simulink model "two_adcs_rfsoc_if_calibrator"  (R2021a) (continued)
== system two_adcs_rfsoc_if_calibrator == (continued)
      mask DialogControl t224_DT_adc0_DataSettings Prompt: Data Settings
      mask DialogControl t224_DT_adc0_req_axis_clk_label Prompt: Required AXI4-Stream clock:
      mask DialogControl t224_DT_adc0_MixerSettings Prompt: MIxer Settings
      mask DialogControl t224_DT_adc0_AnalogSettings Prompt: Analog Settings
      mask DialogControl t224_DT_adc1 Prompt: ADC 1
      mask DialogControl t224_DT_adc1_DataSettings Prompt: Data Settings
      mask DialogControl t224_DT_adc1_req_axis_clk_label Prompt: Required AXI4-Stream clock:
      mask DialogControl t224_DT_adc1_MixerSettings Prompt: MIxer Settings
      mask DialogControl t224_DT_adc1_AnalogSettings Prompt: Analog Settings
      mask DialogControl Tile225_container Prompt: Tile 225
      mask DialogControl pll_clocking_config_225 Prompt: Clocking and PLL Configuration
      mask DialogControl t225_QuadTileConfig Prompt: Quad Tile Configuration
      mask DialogControl t225_ADCPair01 Prompt: ADC Pair 0,1
      mask DialogControl t225_QT_adc0 Prompt: ADC 0
      mask DialogControl t225_QT_adc0_DataSettings Prompt: Data Settings
      mask DialogControl t225_QT_adc0_req_axis_clk_label Prompt: Required AXI4-Stream clock:
      mask DialogControl t225_QT_adc0_MixerSettings Prompt: MIxer Settings
      mask DialogControl t225_QT_adc0_AnalogSettings Prompt: Analog Settings
      mask DialogControl t225_QT_adc1 Prompt: ADC 1
      mask DialogControl t225_QT_adc1_DataSettings Prompt: Data Settings
      mask DialogControl t225_QT_adc1_req_axis_clk_label Prompt: Required AXI4-Stream clock:
      mask DialogControl t225_QT_adc1_MixerSettings Prompt: MIxer Settings
      mask DialogControl t225_QT_adc1_AnalogSettings Prompt: Analog Settings
      mask DialogControl t225_ADCPair23 Prompt: ADC Pair 2,3
      mask DialogControl t225_QT_adc2 Prompt: ADC 2
      mask DialogControl t225_QT_adc2_DataSettings Prompt: Data Settings
      mask DialogControl t225_QT_adc2_req_axis_clk_label Prompt: Required AXI4-Stream clock:
      mask DialogControl t225_QT_adc2_MixerSettings Prompt: MIxer Settings
      mask DialogControl t225_QT_adc2_AnalogSettings Prompt: Analog Settings
      mask DialogControl t225_QT_adc3 Prompt: ADC 3
      mask DialogControl t225_QT_adc3_DataSettings Prompt: Data Settings
      mask DialogControl t225_QT_adc3_req_axis_clk_label Prompt: Required AXI4-Stream clock:
      mask DialogControl t225_QT_adc3_MixerSettings Prompt: MIxer Settings
      mask DialogControl t225_QT_adc3_AnalogSettings Prompt: Analog Settings
      mask DialogControl t225_DualTileConfig Prompt: Dual Tile Configuration
      mask DialogControl t225_DT_adc0 Prompt: ADC 0
      mask DialogControl t225_DT_adc0_DataSettings Prompt: Data Settings
      mask DialogControl t225_DT_adc0_req_axis_clk_label Prompt: Required AXI4-Stream clock:
      mask DialogControl t225_DT_adc0_MixerSettings Prompt: MIxer Settings
      mask DialogControl t225_DT_adc0_AnalogSettings Prompt: Analog Settings
      mask DialogControl t225_DT_adc1 Prompt: ADC 1
      mask DialogControl t225_DT_adc1_DataSettings Prompt: Data Settings
      mask DialogControl t225_DT_adc1_req_axis_clk_label Prompt: Required AXI4-Stream clock:
      mask DialogControl t225_DT_adc1_MixerSettings Prompt: MIxer Settings
      mask DialogControl t225_DT_adc1_AnalogSettings Prompt: Analog Settings
      mask DialogControl Tile226_container Prompt: Tile 226
      mask DialogControl pll_clocking_config_226 Prompt: Clocking and PLL Configuration
      mask DialogControl t226_QuadTileConfig Prompt: Quad Tile Configuration
      mask DialogControl t226_ADCPair01 Prompt: ADC Pair 0,1
      mask DialogControl t226_QT_adc0 Prompt: ADC 0
      mask DialogControl t226_QT_adc0_DataSettings Prompt: Data Settings
      mask DialogControl t226_QT_adc0_req_axis_clk_label Prompt: Required AXI4-Stream clock:
      mask DialogControl t226_QT_adc0_MixerSettings Prompt: MIxer Settings
      mask DialogControl t226_QT_adc0_AnalogSettings Prompt: Analog Settings
      mask DialogControl t226_QT_adc1 Prompt: ADC 1
      mask DialogControl t226_QT_adc1_DataSettings Prompt: Data Settings
      mask DialogControl t226_QT_adc1_req_axis_clk_label Prompt: Required AXI4-Stream clock:
      mask DialogControl t226_QT_adc1_MixerSettings Prompt: MIxer Settings
      mask DialogControl t226_QT_adc1_AnalogSettings Prompt: Analog Settings
      mask DialogControl t226_ADCPair23 Prompt: ADC Pair 2,3
      mask DialogControl t226_QT_adc2 Prompt: ADC 2
      mask DialogControl t226_QT_adc2_DataSettings Prompt: Data Settings
      mask DialogControl t226_QT_adc2_req_axis_clk_label Prompt: Required AXI4-Stream clock:
      mask DialogControl t226_QT_adc2_MixerSettings Prompt: MIxer Settings
      mask DialogControl t226_QT_adc2_AnalogSettings Prompt: Analog Settings
      mask DialogControl t226_QT_adc3 Prompt: ADC 3
      mask DialogControl t226_QT_adc3_DataSettings Prompt: Data Settings
      mask DialogControl t226_QT_adc3_req_axis_clk_label Prompt: Required AXI4-Stream clock:
      mask DialogControl t226_QT_adc3_MixerSettings Prompt: MIxer Settings
      mask DialogControl t226_QT_adc3_AnalogSettings Prompt: Analog Settings
      mask DialogControl t226_DualTileConfig Prompt: Dual Tile Configuration
      mask DialogControl t226_DT_adc0 Prompt: ADC 0
      mask DialogControl t226_DT_adc0_DataSettings Prompt: Data Settings
      mask DialogControl t226_DT_adc0_req_axis_clk_label Prompt: Required AXI4-Stream clock:
      mask DialogControl t226_DT_adc0_MixerSettings Prompt: MIxer Settings
      mask DialogControl t226_DT_adc0_AnalogSettings Prompt: Analog Settings
      mask DialogControl t226_DT_adc1 Prompt: ADC 1
      mask DialogControl t226_DT_adc1_DataSettings Prompt: Data Settings
      mask DialogControl t226_DT_adc1_req_axis_clk_label Prompt: Required AXI4-Stream clock:
      mask DialogControl t226_DT_adc1_MixerSettings Prompt: MIxer Settings
      mask DialogControl t226_DT_adc1_AnalogSettings Prompt: Analog Settings
      mask DialogControl Tile227_container Prompt: Tile 227
      mask DialogControl pll_clocking_config_227 Prompt: Clocking and PLL Configuration
      mask DialogControl t227_QuadTileConfig Prompt: Quad Tile Configuration
      mask DialogControl t227_ADCPair01 Prompt: ADC Pair 0,1
      mask DialogControl t227_QT_adc0 Prompt: ADC 0
      mask DialogControl t227_QT_adc0_DataSettings Prompt: Data Settings
      mask DialogControl t227_QT_adc0_req_axis_clk_label Prompt: Required AXI4-Stream clock:
      mask DialogControl t227_QT_adc0_MixerSettings Prompt: MIxer Settings
      mask DialogControl t227_QT_adc0_AnalogSettings Prompt: Analog Settings
      mask DialogControl t227_QT_adc1 Prompt: ADC 1
      mask DialogControl t227_QT_adc1_DataSettings Prompt: Data Settings
      mask DialogControl t227_QT_adc1_req_axis_clk_label Prompt: Required AXI4-Stream clock:
      mask DialogControl t227_QT_adc1_MixerSettings Prompt: MIxer Settings
      mask DialogControl t227_QT_adc1_AnalogSettings Prompt: Analog Settings
      mask DialogControl t227_ADCPair23 Prompt: ADC Pair 2,3
      mask DialogControl t227_QT_adc2 Prompt: ADC 2
      mask DialogControl t227_QT_adc2_DataSettings Prompt: Data Settings
      mask DialogControl t227_QT_adc2_req_axis_clk_label Prompt: Required AXI4-Stream clock:
      mask DialogControl t227_QT_adc2_MixerSettings Prompt: MIxer Settings
      mask DialogControl t227_QT_adc2_AnalogSettings Prompt: Analog Settings
      mask DialogControl t227_QT_adc3 Prompt: ADC 3
      mask DialogControl t227_QT_adc3_DataSettings Prompt: Data Settings
      mask DialogControl t227_QT_adc3_req_axis_clk_label Prompt: Required AXI4-Stream clock:
      mask DialogControl t227_QT_adc3_MixerSettings Prompt: MIxer Settings
      mask DialogControl t227_QT_adc3_AnalogSettings Prompt: Analog Settings
      mask DialogControl t227_DualTileConfig Prompt: Dual Tile Configuration
      mask DialogControl t227_DT_adc0 Prompt: ADC 0
      mask DialogControl t227_DT_adc0_DataSettings Prompt: Data Settings
      mask DialogControl t227_DT_adc0_req_axis_clk_label Prompt: Required AXI4-Stream clock:
      mask DialogControl t227_DT_adc0_MixerSettings Prompt: MIxer Settings
      mask DialogControl t227_DT_adc0_AnalogSettings Prompt: Analog Settings
      mask DialogControl t227_DT_adc1 Prompt: ADC 1
      mask DialogControl t227_DT_adc1_DataSettings Prompt: Data Settings
      mask DialogControl t227_DT_adc1_req_axis_clk_label Prompt: Required AXI4-Stream clock:
      mask DialogControl t227_DT_adc1_MixerSettings Prompt: MIxer Settings
      mask DialogControl t227_DT_adc1_AnalogSettings Prompt: Analog Settings
      mask DialogControl DACTileSelect Prompt: DAC Tiles
      mask DialogControl Tile228_container Prompt: Tile 228
      mask DialogControl pll_clocking_config_228 Prompt: Clocking and PLL Configuration
      mask DialogControl t228_QuadTileConfig Prompt: Quad Tile Configuration
      mask DialogControl t228_DACPair01 Prompt: DAC Pair 0,1
      mask DialogControl t228_QT_dac0 Prompt: DAC 0
      mask DialogControl t228_QT_dac0_DataSettings Prompt: Data Settings
      mask DialogControl t228_QT_dac0_req_axis_clk_label Prompt: Required AXI4-Stream clock:
      mask DialogControl t228_QT_dac0_MixerSettings Prompt: MIxer Settings
      mask DialogControl t228_QT_dac0_AnalogSettings Prompt: Analog Settings
      mask DialogControl t228_QT_dac1 Prompt: DAC 1
      mask DialogControl t228_QT_dac1_DataSettings Prompt: Data Settings
      mask DialogControl t228_QT_dac1_req_axis_clk_label Prompt: Required AXI4-Stream clock:
      mask DialogControl t228_QT_dac1_MixerSettings Prompt: MIxer Settings
      mask DialogControl t228_QT_dac1_AnalogSettings Prompt: Analog Settings
      mask DialogControl t228_DACPair23 Prompt: DAC Pair 2,3
      mask DialogControl t228_QT_dac2 Prompt: DAC 2
      mask DialogControl t228_QT_dac2_DataSettings Prompt: Data Settings
      mask DialogControl t228_QT_dac2_req_axis_clk_label Prompt: Required AXI4-Stream clock:
      mask DialogControl t228_QT_dac2_MixerSettings Prompt: MIxer Settings
      mask DialogControl t228_QT_dac2_AnalogSettings Prompt: Analog Settings
      mask DialogControl t228_QT_dac3 Prompt: DAC 3
      mask DialogControl t228_QT_dac3_DataSettings Prompt: Data Settings
      mask DialogControl t228_QT_dac3_req_axis_clk_label Prompt: Required AXI4-Stream clock:
      mask DialogControl t228_QT_dac3_MixerSettings Prompt: MIxer Settings
      mask DialogControl t228_QT_dac3_AnalogSettings Prompt: Analog Settings
      mask DialogControl t228_DualTileConfig Prompt: Dual Tile Configuration
      mask DialogControl t228_DACPair01_DT Prompt: DAC Pair 0,1
      mask DialogControl t228_DT_dac0 Prompt: DAC 0
      mask DialogControl t228_DT_dac0_DataSettings Prompt: Data Settings
      mask DialogControl t228_DT_dac0_req_axis_clk_label Prompt: Required AXI4-Stream clock:
      mask DialogControl t228_DT_dac0_MixerSettings Prompt: MIxer Settings
      mask DialogControl t228_DT_dac0_AnalogSettings Prompt: Analog Settings
      mask DialogControl t228_DT_dac1 Prompt: DAC 1
      mask DialogControl t228_DT_dac1_DataSettings Prompt: Data Settings
      mask DialogControl t228_DT_dac1_req_axis_clk_label Prompt: Required AXI4-Stream clock:
      mask DialogControl t228_DT_dac1_MixerSettings Prompt: MIxer Settings
      mask DialogControl t228_DT_dac1_AnalogSettings Prompt: Analog Settings
      mask DialogControl t228_DACPair23_DT Prompt: DAC Pair 2,3
      mask DialogControl t228_DT_dac2 Prompt: DAC 2
      mask DialogControl t228_DT_dac2_DataSettings Prompt: Data Settings
      mask DialogControl t228_DT_dac2_req_axis_clk_label Prompt: Required AXI4-Stream clock:
      mask DialogControl t228_DT_dac2_MixerSettings Prompt: MIxer Settings
      mask DialogControl t228_DT_dac2_AnalogSettings Prompt: Analog Settings
      mask DialogControl t228_DT_dac3 Prompt: DAC 3
      mask DialogControl t228_DT_dac3_DataSettings Prompt: Data Settings
      mask DialogControl t228_DT_dac3_req_axis_clk_label Prompt: Required AXI4-Stream clock:
      mask DialogControl t228_DT_dac3_MixerSettings Prompt: MIxer Settings
      mask DialogControl t228_DT_dac3_AnalogSettings Prompt: Analog Settings
      mask DialogControl Tile229_container Prompt: Tile 229
      mask DialogControl pll_clocking_config_229 Prompt: Clocking and PLL Configuration
      mask DialogControl t229_QuadTileConfig Prompt: Quad Tile Configuration
      mask DialogControl t229_DACPair01 Prompt: DAC Pair 0,1
      mask DialogControl t229_QT_dac0 Prompt: DAC 0
      mask DialogControl t229_QT_dac0_DataSettings Prompt: Data Settings
      mask DialogControl t229_QT_dac0_req_axis_clk_label Prompt: Required AXI4-Stream clock:
      mask DialogControl t229_QT_dac0_MixerSettings Prompt: MIxer Settings
      mask DialogControl t229_QT_dac0_AnalogSettings Prompt: Analog Settings
      mask DialogControl t229_QT_dac1 Prompt: DAC 1
      mask DialogControl t229_QT_dac1_DataSettings Prompt: Data Settings
      mask DialogControl t229_QT_dac1_req_axis_clk_label Prompt: Required AXI4-Stream clock:
      mask DialogControl t229_QT_dac1_MixerSettings Prompt: MIxer Settings
      mask DialogControl t229_QT_dac1_AnalogSettings Prompt: Analog Settings
      mask DialogControl t229_DACPair23 Prompt: DAC Pair 2,3
      mask DialogControl t229_QT_dac2 Prompt: DAC 2
      mask DialogControl t229_QT_dac2_DataSettings Prompt: Data Settings
      mask DialogControl t229_QT_dac2_req_axis_clk_label Prompt: Required AXI4-Stream clock:
      mask DialogControl t229_QT_dac2_MixerSettings Prompt: MIxer Settings
      mask DialogControl t229_QT_dac2_AnalogSettings Prompt: Analog Settings
      mask DialogControl t229_QT_dac3 Prompt: DAC 3
      mask DialogControl t229_QT_dac3_DataSettings Prompt: Data Settings
      mask DialogControl t229_QT_dac3_req_axis_clk_label Prompt: Required AXI4-Stream clock:
      mask DialogControl t229_QT_dac3_MixerSettings Prompt: MIxer Settings
      mask DialogControl t229_QT_dac3_AnalogSettings Prompt: Analog Settings
      mask DialogControl t229_DualTileConfig Prompt: Dual Tile Configuration
      mask DialogControl t229_DACPair01_DT Prompt: DAC Pair 0,1
      mask DialogControl t229_DT_dac0 Prompt: DAC 0
      mask DialogControl t229_DT_dac0_DataSettings Prompt: Data Settings
      mask DialogControl t229_DT_dac0_req_axis_clk_label Prompt: Required AXI4-Stream clock:
      mask DialogControl t229_DT_dac0_MixerSettings Prompt: MIxer Settings
      mask DialogControl t229_DT_dac0_AnalogSettings Prompt: Analog Settings
      mask DialogControl t229_DT_dac1 Prompt: DAC 1
      mask DialogControl t229_DT_dac1_DataSettings Prompt: Data Settings
      mask DialogControl t229_DT_dac1_req_axis_clk_label Prompt: Required AXI4-Stream clock:
      mask DialogControl t229_DT_dac1_MixerSettings Prompt: MIxer Settings
      mask DialogControl t229_DT_dac1_AnalogSettings Prompt: Analog Settings
      mask DialogControl t229_DACPair23_DT Prompt: DAC Pair 2,3
      mask DialogControl t229_DT_dac2 Prompt: DAC 2
      mask DialogControl t229_DT_dac2_DataSettings Prompt: Data Settings
      mask DialogControl t229_DT_dac2_req_axis_clk_label Prompt: Required AXI4-Stream clock:
      mask DialogControl t229_DT_dac2_MixerSettings Prompt: MIxer Settings
      mask DialogControl t229_DT_dac2_AnalogSettings Prompt: Analog Settings
      mask DialogControl t229_DT_dac3 Prompt: DAC 3
      mask DialogControl t229_DT_dac3_DataSettings Prompt: Data Settings
      mask DialogControl t229_DT_dac3_req_axis_clk_label Prompt: Required AXI4-Stream clock:
      mask DialogControl t229_DT_dac3_MixerSettings Prompt: MIxer Settings
      mask DialogControl t229_DT_dac3_AnalogSettings Prompt: Analog Settings
      mask DialogControl Tile230_container Prompt: Tile 230
      mask DialogControl pll_clocking_config_230 Prompt: Clocking and PLL Configuration
      mask DialogControl t230_QuadTileConfig Prompt: Quad Tile Configuration
      mask DialogControl t230_DACPair01 Prompt: DAC Pair 0,1
      mask DialogControl t230_QT_dac0 Prompt: DAC 0
      mask DialogControl t230_QT_dac0_DataSettings Prompt: Data Settings
      mask DialogControl t230_QT_dac0_req_axis_clk_label Prompt: Required AXI4-Stream clock:
      mask DialogControl t230_QT_dac0_MixerSettings Prompt: MIxer Settings
      mask DialogControl t230_QT_dac0_AnalogSettings Prompt: Analog Settings
      mask DialogControl t230_QT_dac1 Prompt: DAC 1
      mask DialogControl t230_QT_dac1_DataSettings Prompt: Data Settings
      mask DialogControl t230_QT_dac1_req_axis_clk_label Prompt: Required AXI4-Stream clock:
      mask DialogControl t230_QT_dac1_MixerSettings Prompt: MIxer Settings
      mask DialogControl t230_QT_dac1_AnalogSettings Prompt: Analog Settings
      mask DialogControl t230_DACPair23 Prompt: DAC Pair 2,3
      mask DialogControl t230_QT_dac2 Prompt: DAC 2
      mask DialogControl t230_QT_dac2_DataSettings Prompt: Data Settings
      mask DialogControl t230_QT_dac2_req_axis_clk_label Prompt: Required AXI4-Stream clock:
      mask DialogControl t230_QT_dac2_MixerSettings Prompt: MIxer Settings
      mask DialogControl t230_QT_dac2_AnalogSettings Prompt: Analog Settings
      mask DialogControl t230_QT_dac3 Prompt: DAC 3
      mask DialogControl t230_QT_dac3_DataSettings Prompt: Data Settings
      mask DialogControl t230_QT_dac3_req_axis_clk_label Prompt: Required AXI4-Stream clock:
      mask DialogControl t230_QT_dac3_MixerSettings Prompt: MIxer Settings
      mask DialogControl t230_QT_dac3_AnalogSettings Prompt: Analog Settings
      mask DialogControl t230_DualTileConfig Prompt: Dual Tile Configuration
      mask DialogControl Control58 Prompt: Tile 230 does not exist for DT configs
      mask DialogControl Tile231_container Prompt: Tile 231
      mask DialogControl pll_clocking_config_231 Prompt: Clocking and PLL Configuration
      mask DialogControl t231_QuadTileConfig Prompt: Quad Tile Configuration
      mask DialogControl t231_DACPair01 Prompt: DAC Pair 0,1
      mask DialogControl t231_QT_dac0 Prompt: DAC 0
      mask DialogControl t231_QT_dac0_DataSettings Prompt: Data Settings
      mask DialogControl t231_QT_dac0_req_axis_clk_label Prompt: Required AXI4-Stream clock:
      mask DialogControl t231_QT_dac0_MixerSettings Prompt: MIxer Settings
      mask DialogControl t231_QT_dac0_AnalogSettings Prompt: Analog Settings
      mask DialogControl t231_QT_dac1 Prompt: DAC 1
      mask DialogControl t231_QT_dac1_DataSettings Prompt: Data Settings
      mask DialogControl t231_QT_dac1_req_axis_clk_label Prompt: Required AXI4-Stream clock:
      mask DialogControl t231_QT_dac1_MixerSettings Prompt: MIxer Settings
      mask DialogControl t231_QT_dac1_AnalogSettings Prompt: Analog Settings
      mask DialogControl t231_DACPair23 Prompt: DAC Pair 2,3
      mask DialogControl t231_QT_dac2 Prompt: DAC 2
      mask DialogControl t231_QT_dac2_DataSettings Prompt: Data Settings
      mask DialogControl t231_QT_dac2_req_axis_clk_label Prompt: Required AXI4-Stream clock:
      mask DialogControl t231_QT_dac2_MixerSettings Prompt: MIxer Settings
      mask DialogControl t231_QT_dac2_AnalogSettings Prompt: Analog Settings
      mask DialogControl t231_QT_dac3 Prompt: DAC 3
      mask DialogControl t231_QT_dac3_DataSettings Prompt: Data Settings
      mask DialogControl t231_QT_dac3_req_axis_clk_label Prompt: Required AXI4-Stream clock:
      mask DialogControl t231_QT_dac3_MixerSettings Prompt: MIxer Settings
      mask DialogControl t231_QT_dac3_AnalogSettings Prompt: Analog Settings
      mask DialogControl t231_DualTileConfig Prompt: Dual Tile Configuration
      mask DialogControl Control59 Prompt: Tile 231 does not exist for DT configs
      mask DialogControl SystemClocking Prompt: System Clocking
      mask DialogControl ADCClocking Prompt: ADC Tile Clocking
      mask DialogControl t224_clock_config Prompt: ADC Tile 0
      mask DialogControl t225_clock_config Prompt: ADC Tile 1
      mask DialogControl t226_clock_config Prompt: ADC Tile 2
      mask DialogControl t227_clock_config Prompt: ADC Tile 3
      mask DialogControl DACClocking Prompt: DAC Tile Clocking
      mask DialogControl t228_clock_config Prompt: DAC Tile 0
      mask DialogControl t229_clock_config Prompt: DAC Tile 1
      mask DialogControl t230_clock_config Prompt: DAC Tile 2
      mask DialogControl t231_clock_config Prompt: DAC Tile 3
  snap_trig : Subsystem  Tag=xps:sw_reg  AncestorBlock=xps_library/Memory/software_register  mask(swreg){io_dir=From Processor, io_delay=0, init_val=dec2hex(0), sample_period=1, names=reg, bitwidths=32, bin_pts=0, arith_types=2, sim_port=off, show_format=on}
      mask prompts: io_dir "I/O direction"; io_delay "I/O delay"; init_val "Initial Value"; sample_period "Sample period"; names "Bitfield names [msb...lsb]"; bitwidths "Bitfield widths"; bin_pts "Bitfield binary pts"; arith_types "Bitfield types, ufix=0, fix=1, bool=2"; sim_port "Provide sim input/output?"; show_format "Print format string?"
      mask help: A 32-bit software-accessible register. Can be divided into bitfields of varying widths and types using the fields in the block mask.
      mask init:
      | swreg_init(gcb)
      settings: AttributesFormatString=1input:b = 32 bits
      mask TypeOptions io_dir: To Processor | From Processor
      mask Callback io_dir:
      | mask_enables = get_param(gcb, 'MaskEnables');
      | mask_names = get_param(gcb, 'MaskNames');
      | if strcmp(get_param(gcb, 'io_dir'), 'To Processor'),
      |     mask_enables{ismember(mask_names, 'sample_period')} = 'off';
      | else
      |     mask_enables{ismember(mask_names, 'sample_period')} = 'on';
      | end
      | set_param(gcb, 'MaskEnables', mask_enables);
      mask DialogControl ParameterTabVar0 Prompt: Setup
  sync_gen : Subsystem  AncestorBlock=casper_library_misc/sync_gen  mask(sync_gen){sim_acc_len=1, fft_size=2048, fft_simult_inputs=8, pfb_fir_taps=3, reorder_vec=[2,2,8], scale=4096}
      mask prompts: sim_acc_len "Simulation Accumulation Length"; fft_size "FFT Size"; fft_simult_inputs "Simultaneous Inputs (FFT)"; pfb_fir_taps "Taps in pfb_fir (use 1 if no pfb_fir)"; reorder_vec "Reorder Orders (e.g. [2 2 2])"
      mask help:
      | This block computes the sync period based on system parameters as
      | described in Casper memo.  Detailed descritions of the block's
      | variables can be found in the 'Block Documentation' section of
      | the CASPER wiki.
      mask init:
      | sync_gen_init(gcb, ...
      |     'sim_acc_len', sim_acc_len, ...
      |     'fft_size', fft_size, ...
      |     'fft_simult_inputs', fft_simult_inputs,...
      |     'pfb_fir_taps', pfb_fir_taps, ...
      |     'reorder_vec', reorder_vec, ...
      |     'scale', scale);
      settings: AttributesFormatString=sim_sync_period=25165824
      mask Help:
      | SyncPeriod=LCM(reorder_vec)*(acc_num)*(pfb_fir_len)*(fft_size/fft_simult_inputs)
      | 
      | See CASPER sync pulse memo for more details.
  wires:
  Constant2/1 -> rfdc/1(m00_axis_tdata_sim), rfdc/2(m01_axis_tdata_sim), rfdc/3(m02_axis_tdata_sim), rfdc/4(m03_axis_tdata_sim), rfdc/5(m10_axis_tdata_sim), rfdc/6(m12_axis_tdata_sim), rfdc/8(m21_axis_tdata_sim), rfdc/12(m32_axis_tdata_sim), rfdc/11(m30_axis_tdata_sim), rfdc/10(m23_axis_tdata_sim), rfdc/9(m22_axis_tdata_sim), rfdc/7(m20_axis_tdata_sim)
  snap_trig/1(in_reg) -> [snap_trig]
  pfb_fir0/1(sync_out) -> fft0/1(sync)
  pfb_fir0/2(pol1_out1) -> fft0/3(in00)
  pfb_fir0/3(pol1_out2) -> fft0/4(in01)
  pfb_fir0/4(pol1_out3) -> fft0/5(in02)
  pfb_fir0/5(pol1_out4) -> fft0/6(in03)
  pfb_fir0/6(pol1_out5) -> fft0/7(in04)
  pfb_fir0/7(pol1_out6) -> fft0/8(in05)
  pfb_fir0/8(pol1_out7) -> fft0/9(in06)
  pfb_fir0/9(pol1_out8) -> fft0/10(in07)
  pre_power_delay0/2(dout0) -> pre_correlation_delay0/2(din0), power0/2(din0), debug_sys/3(fft0_0), calibrator_1/2(fft0_0), calibrator_0/2(fft0_0)
  pre_power_delay0/3(dout1) -> pre_correlation_delay0/3(din1), power0/3(din1), calibrator_1/3(fft0_1), calibrator_0/3(fft0_1)
  pre_power_delay0/4(dout2) -> calibrator_1/4(fft0_2), calibrator_0/4(fft0_2), pre_correlation_delay0/4(din2), power0/4(din2)
  pre_power_delay0/5(dout3) -> calibrator_1/5(fft0_3), calibrator_0/5(fft0_3), pre_correlation_delay0/5(din3), power0/5(din3)
  [cnt_rst] -> acc_cntrl0/3(rst)
  [acc_len_cal] -> acc_cntrl0/1(acc_len)
  post_power_delay0/1(sync_out) -> acc_cntrl0/2(sync)
  pipeline8/1(q) -> [acc_len_cal]
  acc_len_cal/1(in_reg) -> pipeline8/1(d)
  pipeline1/1(q) -> [cnt_rst]
  cnt_rst/1(in_reg) -> pipeline1/1(d)
  acc_cntrl0/1(new_acc) -> counter0/1, accs0/1(new_acc)
  post_power_delay0/2(dout0) -> Delay/1
  post_power_delay0/3(dout1) -> Delay1/1
  post_power_delay0/5(dout3) -> Delay3/1
  post_power_delay0/4(dout2) -> Delay2/1
  Delay/1 -> accs0/2(din)
  Delay1/1 -> accs0/3(din1)
  Delay2/1 -> accs0/4(din2)
  Delay3/1 -> accs0/5(din3)
  accs0/1(valid) -> counter0/2, pre_bram_delay0/2(valid_in)
  accs0/2(dout0) -> pre_bram_delay0/3(din0)
  accs0/3(dout1) -> pre_bram_delay0/4(din1)
  accs0/4(dout2) -> pre_bram_delay0/5(din2)
  accs0/5(dout3) -> pre_bram_delay0/6(din3)
  counter0/1 -> pre_bram_delay0/1(addr_in)
  pre_bram_delay0/1(addr_out) -> adc0_0/1(addr), adc0_1/1(addr), adc0_2/1(addr), adc0_7/1(addr), adc0_6/1(addr), adc0_5/1(addr), adc0_4/1(addr), adc0_3/1(addr)
  pre_bram_delay0/3(dout0) -> adc0_0/2(data_in)
  pre_bram_delay0/2(valid_out) -> adc0_7/3(we), adc0_6/3(we), adc0_5/3(we), adc0_4/3(we), adc0_3/3(we), adc0_2/3(we), adc0_1/3(we), adc0_0/3(we)
  pre_bram_delay0/4(dout1) -> adc0_1/2(data_in)
  pre_bram_delay0/5(dout2) -> adc0_2/2(data_in)
  pre_bram_delay0/6(dout3) -> adc0_3/2(data_in)
  adc0_0/1(data_out) -> Terminator3/1
  adc0_1/1(data_out) -> Terminator4/1
  adc0_2/1(data_out) -> Terminator5/1
  adc0_3/1(data_out) -> Terminator6/1
  power0/1(sync_out) -> power_convert0/1(sync_in)
  power0/2(dout0) -> power_convert0/2(din0)
  power0/3(dout1) -> power_convert0/3(din1)
  power0/4(dout2) -> power_convert0/4(din2)
  power0/5(dout3) -> power_convert0/5(din3)
  power_convert0/1(sync_out) -> post_power_delay0/1(sync_in)
  power_convert0/2(dout0) -> post_power_delay0/2(din0)
  power_convert0/3(dout1) -> post_power_delay0/3(din1)
  power_convert0/4(dout2) -> post_power_delay0/4(din2)
  power_convert0/5(dout3) -> post_power_delay0/5(din3)
  Constant6/1 -> [fft_shift]
  [fft_shift] -> fft0/2(shift)
  rfdc/1(m00_axis_tdata) -> adcsnap0/1(din_real), bus_create/1(in1)
  rfdc/2(m01_axis_tdata) -> adcsnap0/2(din_imag), bus_create/2(in2)
  bus_create/1(bus_out) -> munge1/1(din)
  munge1/1(dout) -> [m00]
  munge2/1(dout) -> [m02]
  bus_create1/1(bus_out) -> munge2/1(din)
  rfdc/3(m02_axis_tdata) -> bus_create1/1(in1), adcsnap1/1(din_real)
  rfdc/4(m03_axis_tdata) -> adcsnap1/2(din_imag), bus_create1/2(in2)
  bus_create3/1(bus_out) -> munge4/1(din)
  bus_create2/1(bus_out) -> munge3/1(din)
  rfdc/7(m20_axis_tdata) -> bus_create2/1(in1)
  rfdc/9(m22_axis_tdata) -> bus_create3/1(in1)
  rfdc/10(m23_axis_tdata) -> bus_create3/2(in2)
  rfdc/8(m21_axis_tdata) -> bus_create2/2(in2)
  munge3/1(dout) -> [m20]
  fft0/1(sync_out) -> pre_power_delay0/1(sync_in)
  fft0/2(out00) -> pre_power_delay0/2(din0)
  fft0/3(out01) -> pre_power_delay0/3(din1)
  fft0/4(out02) -> pre_power_delay0/4(din2)
  fft0/5(out03) -> pre_power_delay0/5(din3)
  fft0/6(out04) -> pre_power_delay0/6(din4)
  fft0/7(out05) -> pre_power_delay0/7(din5)
  fft0/8(out06) -> pre_power_delay0/8(din6)
  fft0/9(out07) -> pre_power_delay0/9(din7)
  pre_power_delay0/6(dout4) -> calibrator_1/6(fft0_4), calibrator_0/6(fft0_4), pre_correlation_delay0/6(din4), power0/6(din4)
  pre_power_delay0/7(dout5) -> calibrator_1/7(fft0_5), calibrator_0/7(fft0_5), pre_correlation_delay0/7(din5), power0/7(din5)
  pre_power_delay0/8(dout6) -> calibrator_1/8(fft0_6), calibrator_0/8(fft0_6), pre_correlation_delay0/8(din6), power0/8(din6)
  pre_power_delay0/9(dout7) -> calibrator_1/9(fft0_7), calibrator_0/9(fft0_7), pre_correlation_delay0/9(din7), power0/9(din7)
  power0/6(dout4) -> power_convert0/6(din4)
  power0/7(dout5) -> power_convert0/7(din5)
  power0/8(dout6) -> power_convert0/8(din6)
  power0/9(dout7) -> power_convert0/9(din7)
  power_convert0/6(dout4) -> post_power_delay0/6(din4)
  power_convert0/7(dout5) -> post_power_delay0/7(din5)
  power_convert0/8(dout6) -> post_power_delay0/8(din6)
  power_convert0/9(dout7) -> post_power_delay0/9(din7)
  Delay16/1 -> accs0/6(din4)
  post_power_delay0/6(dout4) -> Delay16/1
  post_power_delay0/7(dout5) -> Delay17/1
  post_power_delay0/8(dout6) -> Delay18/1
  post_power_delay0/9(dout7) -> Delay19/1
  Delay17/1 -> accs0/7(din5)
  Delay18/1 -> accs0/8(din6)
  Delay19/1 -> accs0/9(din7)
  accs0/6(dout4) -> pre_bram_delay0/7(din4)
  accs0/7(dout5) -> pre_bram_delay0/8(din5)
  accs0/8(dout6) -> pre_bram_delay0/9(din6)
  accs0/9(dout7) -> pre_bram_delay0/10(din7)
  adc0_7/1(data_out) -> Terminator23/1
  adc0_5/1(data_out) -> Terminator21/1
  adc0_6/1(data_out) -> Terminator22/1
  adc0_4/1(data_out) -> Terminator20/1
  pre_bram_delay0/7(dout4) -> adc0_4/2(data_in)
  pre_bram_delay0/8(dout5) -> adc0_5/2(data_in)
  pre_bram_delay0/9(dout6) -> adc0_6/2(data_in)
  pre_bram_delay0/10(dout7) -> adc0_7/2(data_in)
  pfb_fir1/4(pol1_out3) -> fft1/5(in02)
  fft1/7(out05) -> pre_power_delay1/7(din5)
  fft1/4(out02) -> pre_power_delay1/4(din2)
  pfb_fir1/5(pol1_out4) -> fft1/6(in03)
  pre_power_delay1/4(dout2) -> calibrator_1/12(fft1_2), calibrator_0/12(fft1_2), power1/4(din2), conjugation0/3(z2)
  pfb_fir1/1(sync_out) -> fft1/1(sync)
  pre_power_delay1/9(dout7) -> calibrator_1/17(fft1_7), calibrator_0/17(fft1_7), power1/9(din7), conjugation0/8(z7)
  pre_power_delay1/6(dout4) -> calibrator_1/14(fft1_4), calibrator_0/14(fft1_4), power1/6(din4), conjugation0/5(z4)
  pre_power_delay1/3(dout1) -> calibrator_0/11(fft1_1), calibrator_1/11(fft1_1), power1/3(din1), conjugation0/2(z1)
  fft1/5(out03) -> pre_power_delay1/5(din3)
  fft1/1(sync_out) -> pre_power_delay1/1(sync_in)
  pfb_fir1/9(pol1_out8) -> fft1/10(in07)
  pfb_fir1/6(pol1_out5) -> fft1/7(in04)
  fft1/2(out00) -> pre_power_delay1/2(din0)
  pfb_fir1/8(pol1_out7) -> fft1/9(in06)
  fft1/8(out06) -> pre_power_delay1/8(din6)
  pre_power_delay1/5(dout3) -> calibrator_1/13(fft1_3), calibrator_0/13(fft1_3), power1/5(din3), conjugation0/4(z3)
  pre_power_delay1/8(dout6) -> calibrator_1/16(fft1_6), calibrator_0/16(fft1_6), power1/8(din6), conjugation0/7(z6)
  pre_power_delay1/2(dout0) -> power1/2(din0), conjugation0/1(z), debug_sys/4(fft1_0), calibrator_1/10(fft1_0), calibrator_0/10(fft1_0)
  pfb_fir1/3(pol1_out2) -> fft1/4(in01)
  fft1/9(out07) -> pre_power_delay1/9(din7)
  pfb_fir1/2(pol1_out1) -> fft1/3(in00)
  pre_power_delay1/7(dout5) -> calibrator_1/15(fft1_5), calibrator_0/15(fft1_5), power1/7(din5), conjugation0/6(z5)
  fft1/6(out04) -> pre_power_delay1/6(din4)
  fft1/3(out01) -> pre_power_delay1/3(din1)
  pre_power_delay1/1(sync_out) -> power1/1(sync_in)
  pfb_fir1/7(pol1_out6) -> fft1/8(in05)
  [fft_shift] -> fft1/2(shift)
  munge4/1(dout) -> [m22]
  pre_correlation_delay0/1(sync_out) -> correlation_mults0/1(sync_in)
  pre_correlation_delay0/2(dout0) -> correlation_mults0/2(adc0_0)
  pre_correlation_delay0/3(dout1) -> correlation_mults0/3(adc0_1)
  pre_correlation_delay0/4(dout2) -> correlation_mults0/4(adc0_2)
  pre_correlation_delay0/5(dout3) -> correlation_mults0/5(adc0_3)
  pre_correlation_delay0/6(dout4) -> correlation_mults0/6(adc0_4)
  pre_correlation_delay0/7(dout5) -> correlation_mults0/7(adc0_5)
  pre_correlation_delay0/8(dout6) -> correlation_mults0/8(adc0_6)
  pre_correlation_delay0/9(dout7) -> correlation_mults0/9(adc0_7)
  conjugation0/1(z*) -> correlation_mults0/10(adc1_0)
  conjugation0/2(z*1) -> correlation_mults0/11(adc1_1)
  conjugation0/3(z*2) -> correlation_mults0/12(adc1_2)
  conjugation0/4(z*3) -> correlation_mults0/13(adc1_3)
  conjugation0/5(z*4) -> correlation_mults0/14(adc1_4)
  conjugation0/6(z*5) -> correlation_mults0/15(adc1_5)
  conjugation0/7(z*6) -> correlation_mults0/16(adc1_6)
  conjugation0/8(z*7) -> correlation_mults0/17(adc1_7)
  correlation_mults0/4(corr2) -> correlation_conv0/4(din2)
  correlation_mults0/3(corr1) -> correlation_conv0/3(din1)
  correlation_mults0/5(corr3) -> correlation_conv0/5(din3)
  correlation_mults0/2(corr0) -> correlation_conv0/2(din0)
  correlation_mults0/6(corr4) -> correlation_conv0/6(din4)
  correlation_mults0/1(sync_out) -> correlation_conv0/1(sync_in)
  correlation_mults0/7(corr5) -> correlation_conv0/7(din5)
  correlation_mults0/8(corr6) -> correlation_conv0/8(din6)
  correlation_mults0/9(corr7) -> correlation_conv0/9(din7)
  correlation_conv0/1(sync_out) -> post_correlation_delay0/1(sync_in)
  correlation_conv0/2(dout0) -> post_correlation_delay0/2(din0)
  correlation_conv0/3(dout1) -> post_correlation_delay0/3(din1)
  correlation_conv0/4(dout2) -> post_correlation_delay0/4(din2)
  correlation_conv0/5(dout3) -> post_correlation_delay0/5(din3)
  correlation_conv0/6(dout4) -> post_correlation_delay0/6(din4)
  correlation_conv0/7(dout5) -> post_correlation_delay0/7(din5)
  correlation_conv0/8(dout6) -> post_correlation_delay0/8(din6)
  correlation_conv0/9(dout7) -> post_correlation_delay0/9(din7)
  correlation_accs0/8(dout6) -> pre_bram_delay4/9(din6)
  Delay36/1 -> correlation_accs0/8(din6)
  post_correlation_delay0/3(dout1) -> Delay33/1
  counter4/1 -> pre_bram_delay4/1(addr_in)
  Delay33/1 -> correlation_accs0/3(din1)
  post_correlation_delay0/2(dout0) -> Delay32/1
  correlation_accs0/1(valid) -> counter4/2, pre_bram_delay4/2(valid_in)
  Delay34/1 -> correlation_accs0/6(din4)
  correlation_accs0/9(dout7) -> pre_bram_delay4/10(din7)
  post_correlation_delay0/5(dout3) -> Delay39/1
  correlation_accs0/2(dout0) -> pre_bram_delay4/3(din0)
  correlation_accs0/5(dout3) -> pre_bram_delay4/6(din3)
  post_correlation_delay0/8(dout6) -> Delay36/1
  post_correlation_delay0/9(dout7) -> Delay37/1
  post_correlation_delay0/1(sync_out) -> acc_cntrl4/2(sync)
  [cnt_rst] -> acc_cntrl4/3(rst)
  correlation_accs0/6(dout4) -> pre_bram_delay4/7(din4)
  Delay37/1 -> correlation_accs0/9(din7)
  correlation_accs0/4(dout2) -> pre_bram_delay4/5(din2)
  correlation_accs0/3(dout1) -> pre_bram_delay4/4(din1)
  post_correlation_delay0/7(dout5) -> Delay35/1
  correlation_accs0/7(dout5) -> pre_bram_delay4/8(din5)
  [acc_len_cal] -> acc_cntrl4/1(acc_len)
  post_correlation_delay0/6(dout4) -> Delay34/1
  post_correlation_delay0/4(dout2) -> Delay38/1
  Delay32/1 -> correlation_accs0/2(din)
  Delay38/1 -> correlation_accs0/4(din2)
  Delay39/1 -> correlation_accs0/5(din3)
  Delay35/1 -> correlation_accs0/7(din5)
  acc_cntrl4/1(new_acc) -> counter4/1, correlation_accs0/1(new_acc)
  pre_bram_delay4/1(addr_out) -> corr01_2/1(addr), corr01_4/1(addr), corr01_6/1(addr), corr01_7/1(addr), corr01_5/1(addr), corr01_3/1(addr), corr01_1/1(addr), corr01_0/1(addr)
  pre_bram_delay4/3(dout0) -> corr01_0/2(data_in)
  pre_bram_delay4/2(valid_out) -> corr01_1/3(we), corr01_2/3(we), corr01_4/3(we), corr01_5/3(we), corr01_6/3(we), corr01_7/3(we), corr01_3/3(we), corr01_0/3(we)
  pre_bram_delay4/4(dout1) -> corr01_1/2(data_in)
  pre_bram_delay4/5(dout2) -> corr01_2/2(data_in)
  pre_bram_delay4/6(dout3) -> corr01_3/2(data_in)
  pre_bram_delay4/7(dout4) -> corr01_4/2(data_in)
  pre_bram_delay4/8(dout5) -> corr01_5/2(data_in)
  pre_bram_delay4/9(dout6) -> corr01_6/2(data_in)
  pre_bram_delay4/10(dout7) -> corr01_7/2(data_in)
  pipeline2/1(q) -> [acc_len_synth]
  acc_len_synth/1(in_reg) -> pipeline2/1(d)
  [snap_trig] -> adcsnap0/4(trig)
  Constant4/1 -> adcsnap0/3(we)
  Constant1/1 -> adcsnap1/3(we)
  [snap_trig] -> adcsnap1/4(trig)
  sync_gen/1(sync_out) -> [sync]
  [sync] -> delay_wideband_prog1/1(sync)
  pipeline3/1(q) -> [adc0_delay]
  adc0_delay/1(in_reg) -> pipeline3/1(d)
  pipeline4/1(q) -> [adc1_delay]
  adc1_delay/1(in_reg) -> pipeline4/1(d)
  [adc0_delay] -> delay_wideband_prog1/2(delay)
  Constant3/1 -> delay_wideband_prog1/3(ld_delay)
  bus_expand/1(msb_out8) -> delay_wideband_prog1/4(data_in0)
  bus_expand/2(out7) -> delay_wideband_prog1/5(data_in1)
  bus_expand/3(out6) -> delay_wideband_prog1/6(data_in2)
  bus_expand/4(out5) -> delay_wideband_prog1/7(data_in3)
  bus_expand/5(out4) -> delay_wideband_prog1/8(data_in4)
  bus_expand/6(out3) -> delay_wideband_prog1/9(data_in5)
  bus_expand/7(out2) -> delay_wideband_prog1/10(data_in6)
  bus_expand/8(lsb_out1) -> delay_wideband_prog1/11(data_in7)
  delay_wideband_prog1/1(sync_out) -> pfb_fir0/1(sync)
  delay_wideband_prog1/2(data_out0) -> pfb_fir0/2(pol1_in1)
  delay_wideband_prog1/3(data_out1) -> pfb_fir0/3(pol1_in2)
  delay_wideband_prog1/4(data_out2) -> pfb_fir0/4(pol1_in3)
  delay_wideband_prog1/5(data_out3) -> pfb_fir0/5(pol1_in4)
  delay_wideband_prog1/6(data_out4) -> pfb_fir0/6(pol1_in5)
  delay_wideband_prog1/7(data_out5) -> pfb_fir0/7(pol1_in6)
  delay_wideband_prog1/8(data_out6) -> pfb_fir0/8(pol1_in7)
  delay_wideband_prog1/9(data_out7) -> pfb_fir0/9(pol1_in8)
  [m00] -> bus_expand/1(bus_in)
  Constant5/1 -> delay_wideband_prog2/3(ld_delay)
  bus_expand1/2(out7) -> delay_wideband_prog2/5(data_in1)
  bus_expand1/4(out5) -> delay_wideband_prog2/7(data_in3)
  [m02] -> bus_expand1/1(bus_in)
  bus_expand1/1(msb_out8) -> delay_wideband_prog2/4(data_in0)
  [adc1_delay] -> delay_wideband_prog2/2(delay)
  bus_expand1/8(lsb_out1) -> delay_wideband_prog2/11(data_in7)
  [sync] -> delay_wideband_prog2/1(sync)
  bus_expand1/3(out6) -> delay_wideband_prog2/6(data_in2)
  bus_expand1/7(out2) -> delay_wideband_prog2/10(data_in6)
  bus_expand1/5(out4) -> delay_wideband_prog2/8(data_in4)
  bus_expand1/6(out3) -> delay_wideband_prog2/9(data_in5)
  delay_wideband_prog2/1(sync_out) -> pfb_fir1/1(sync)
  delay_wideband_prog2/2(data_out0) -> pfb_fir1/2(pol1_in1)
  delay_wideband_prog2/3(data_out1) -> pfb_fir1/3(pol1_in2)
  delay_wideband_prog2/4(data_out2) -> pfb_fir1/4(pol1_in3)
  delay_wideband_prog2/5(data_out3) -> pfb_fir1/5(pol1_in4)
  delay_wideband_prog2/6(data_out4) -> pfb_fir1/6(pol1_in5)
  delay_wideband_prog2/7(data_out5) -> pfb_fir1/7(pol1_in6)
  delay_wideband_prog2/8(data_out6) -> pfb_fir1/8(pol1_in7)
  delay_wideband_prog2/9(data_out7) -> pfb_fir1/9(pol1_in8)
  calibrator_1/5(dout3) -> calibrator_conv1/5(din3)
  calibrator_conv1/8(dout6) -> calibrator_delay1/8(din6)
  pre_bram_delay3/3(dout0) -> cal1_0/2(data_in)
  calibrator_accs1/6(dout4) -> pre_bram_delay3/7(din4)
  pre_bram_delay3/2(valid_out) -> cal1_4/3(we), cal1_5/3(we), cal1_7/3(we), cal1_6/3(we), cal1_3/3(we), cal1_2/3(we), cal1_1/3(we), cal1_0/3(we)
  calibrator_1/6(dout4) -> calibrator_conv1/6(din4)
  calibrator_conv1/2(dout0) -> calibrator_delay1/2(din0)
  acc_cntrl3/1(new_acc) -> calibrator_accs1/1(new_acc), counter3/1
  calibrator_accs1/9(dout7) -> pre_bram_delay3/10(din7)
  calibrator_delay1/3(dout1) -> Delay25/1
  pre_bram_delay3/10(dout7) -> cal1_7/2(data_in)
  calibrator_delay1/4(dout2) -> Delay30/1
  calibrator_accs1/5(dout3) -> pre_bram_delay3/6(din3)
  Delay27/1 -> calibrator_accs1/7(din5)
  calibrator_accs1/4(dout2) -> pre_bram_delay3/5(din2)
  Delay24/1 -> calibrator_accs1/2(din)
  calibrator_delay1/8(dout6) -> Delay28/1
  pre_bram_delay3/7(dout4) -> cal1_4/2(data_in)
  calibrator_1/7(dout5) -> calibrator_conv1/7(din5)
  calibrator_1/4(dout2) -> calibrator_conv1/4(din2)
  Delay31/1 -> calibrator_accs1/5(din3)
  calibrator_conv1/4(dout2) -> calibrator_delay1/4(din2)
  calibrator_conv1/3(dout1) -> calibrator_delay1/3(din1)
  calibrator_1/8(dout6) -> calibrator_conv1/8(din6)
  pre_bram_delay3/4(dout1) -> cal1_1/2(data_in)
  calibrator_conv1/6(dout4) -> calibrator_delay1/6(din4)
  calibrator_conv1/7(dout5) -> calibrator_delay1/7(din5)
  pre_bram_delay3/9(dout6) -> cal1_6/2(data_in)
  calibrator_delay1/2(dout0) -> Delay24/1
  calibrator_delay1/7(dout5) -> Delay27/1
  pre_bram_delay3/5(dout2) -> cal1_2/2(data_in)
  calibrator_delay1/1(sync_out) -> acc_cntrl3/2(sync)
  calibrator_accs1/7(dout5) -> pre_bram_delay3/8(din5)
  pre_bram_delay3/6(dout3) -> cal1_3/2(data_in)
  calibrator_conv1/5(dout3) -> calibrator_delay1/5(din3)
  Delay28/1 -> calibrator_accs1/8(din6)
  calibrator_delay1/9(dout7) -> Delay29/1
  calibrator_conv1/9(dout7) -> calibrator_delay1/9(din7)
  calibrator_delay1/6(dout4) -> Delay26/1
  calibrator_1/9(dout7) -> calibrator_conv1/9(din7)
  calibrator_1/1(sync_out) -> calibrator_conv1/1(sync_in)
  calibrator_conv1/1(sync_out) -> calibrator_delay1/1(sync_in)
  Delay30/1 -> calibrator_accs1/4(din2)
  calibrator_accs1/3(dout1) -> pre_bram_delay3/4(din1)
  counter3/1 -> pre_bram_delay3/1(addr_in)
  [acc_len_synth] -> acc_cntrl3/1(acc_len)
  calibrator_1/3(dout1) -> calibrator_conv1/3(din1)
  Delay25/1 -> calibrator_accs1/3(din1)
  calibrator_accs1/2(dout0) -> pre_bram_delay3/3(din0)
  calibrator_accs1/8(dout6) -> pre_bram_delay3/9(din6)
  pre_bram_delay3/1(addr_out) -> cal1_4/1(addr), cal1_7/1(addr), cal1_6/1(addr), cal1_5/1(addr), cal1_3/1(addr), cal1_2/1(addr), cal1_1/1(addr), cal1_0/1(addr)
  Delay26/1 -> calibrator_accs1/6(din4)
  [cnt_rst] -> acc_cntrl3/3(rst)
  Delay29/1 -> calibrator_accs1/9(din7)
  calibrator_delay1/5(dout3) -> Delay31/1
  calibrator_accs1/1(valid) -> pre_bram_delay3/2(valid_in), counter3/2
  pre_bram_delay3/8(dout5) -> cal1_5/2(data_in)
  calibrator_1/2(dout0) -> calibrator_conv1/2(din0)
  calibrator_conv0/7(dout5) -> calibrator_delay0/7(din5)
  pre_bram_delay2/10(dout7) -> cal0_7/2(data_in)
  [cnt_rst] -> acc_cntrl2/3(rst)
  calibrator_delay0/3(dout1) -> Delay13/1
  acc_cntrl2/1(new_acc) -> calibrator_accs0/1(new_acc), counter2/1
  calibrator_delay0/7(dout5) -> Delay15/1
  counter2/1 -> pre_bram_delay2/1(addr_in)
  calibrator_0/8(dout6) -> calibrator_conv0/8(din6)
  calibrator_0/3(dout1) -> calibrator_conv0/3(din1)
  Delay13/1 -> calibrator_accs0/3(din1)
  calibrator_delay0/5(dout3) -> Delay23/1
  Delay23/1 -> calibrator_accs0/5(din3)
  calibrator_conv0/3(dout1) -> calibrator_delay0/3(din1)
  calibrator_conv0/2(dout0) -> calibrator_delay0/2(din0)
  pre_bram_delay2/2(valid_out) -> cal0_4/3(we), cal0_5/3(we), cal0_7/3(we), cal0_6/3(we), cal0_3/3(we), cal0_2/3(we), cal0_1/3(we), cal0_0/3(we)
  calibrator_0/4(dout2) -> calibrator_conv0/4(din2)
  calibrator_accs0/5(dout3) -> pre_bram_delay2/6(din3)
  calibrator_0/2(dout0) -> calibrator_conv0/2(din0)
  pre_bram_delay2/8(dout5) -> cal0_5/2(data_in)
  pre_bram_delay2/3(dout0) -> cal0_0/2(data_in)
  calibrator_delay0/1(sync_out) -> acc_cntrl2/2(sync)
  calibrator_conv0/4(dout2) -> calibrator_delay0/4(din2)
  pre_bram_delay2/7(dout4) -> cal0_4/2(data_in)
  calibrator_accs0/1(valid) -> pre_bram_delay2/2(valid_in), counter2/2
  calibrator_accs0/7(dout5) -> pre_bram_delay2/8(din5)
  pre_bram_delay2/6(dout3) -> cal0_3/2(data_in)
  calibrator_conv0/6(dout4) -> calibrator_delay0/6(din4)
  pre_bram_delay2/5(dout2) -> cal0_2/2(data_in)
  calibrator_0/6(dout4) -> calibrator_conv0/6(din4)
  pre_bram_delay2/4(dout1) -> cal0_1/2(data_in)
  calibrator_delay0/4(dout2) -> Delay22/1
  Delay12/1 -> calibrator_accs0/2(din)
  calibrator_accs0/2(dout0) -> pre_bram_delay2/3(din0)
  calibrator_delay0/8(dout6) -> Delay20/1
  pre_bram_delay2/1(addr_out) -> cal0_4/1(addr), cal0_7/1(addr), cal0_6/1(addr), cal0_5/1(addr), cal0_3/1(addr), cal0_2/1(addr), cal0_1/1(addr), cal0_0/1(addr)
  Delay22/1 -> calibrator_accs0/4(din2)
  Delay20/1 -> calibrator_accs0/8(din6)
  calibrator_delay0/2(dout0) -> Delay12/1
  Delay15/1 -> calibrator_accs0/7(din5)
  calibrator_0/9(dout7) -> calibrator_conv0/9(din7)
  calibrator_accs0/9(dout7) -> pre_bram_delay2/10(din7)
  calibrator_accs0/6(dout4) -> pre_bram_delay2/7(din4)
  calibrator_conv0/1(sync_out) -> calibrator_delay0/1(sync_in)
  calibrator_0/7(dout5) -> calibrator_conv0/7(din5)
  calibrator_conv0/8(dout6) -> calibrator_delay0/8(din6)
  calibrator_delay0/9(dout7) -> Delay21/1
  calibrator_accs0/8(dout6) -> pre_bram_delay2/9(din6)
  calibrator_0/1(sync_out) -> calibrator_conv0/1(sync_in)
  calibrator_delay0/6(dout4) -> Delay14/1
  calibrator_accs0/3(dout1) -> pre_bram_delay2/4(din1)
  calibrator_conv0/9(dout7) -> calibrator_delay0/9(din7)
  [acc_len_synth] -> acc_cntrl2/1(acc_len)
  calibrator_0/5(dout3) -> calibrator_conv0/5(din3)
  Delay14/1 -> calibrator_accs0/6(din4)
  pre_bram_delay2/9(dout6) -> cal0_6/2(data_in)
  calibrator_accs0/4(dout2) -> pre_bram_delay2/5(din2)
  Delay21/1 -> calibrator_accs0/9(din7)
  calibrator_conv0/5(dout3) -> calibrator_delay0/5(din3)
  pre_power_delay0/1(sync_out) -> power0/1(sync_in), pre_correlation_delay0/1(sync_in), debug_sys/1(sync_in), calibrator_1/1(sync_in), calibrator_0/1(sync_in)
  debug/1(in_reg) -> debug_sys/2(debug)
  power1/2(dout0) -> power_convert1/2(din0)
  [acc_len_cal] -> acc_cntrl1/1(acc_len)
  power1/4(dout2) -> power_convert1/4(din2)
  power_convert1/4(dout2) -> post_power_delay1/4(din2)
  power_convert1/2(dout0) -> post_power_delay1/2(din0)
  pre_bram_delay1/7(dout4) -> adc1_4/2(data_in)
  power1/8(dout6) -> power_convert1/8(din6)
  accs1/9(dout7) -> pre_bram_delay1/10(din7)
  post_power_delay1/2(dout0) -> Delay4/1
  acc_cntrl1/1(new_acc) -> counter1/1, accs1/1(new_acc)
  Delay9/1 -> accs1/9(din7)
  adc1_5/1(data_out) -> Terminator2/1
  accs1/7(dout5) -> pre_bram_delay1/8(din5)
  accs1/4(dout2) -> pre_bram_delay1/5(din2)
  Delay11/1 -> accs1/5(din3)
  Delay4/1 -> accs1/2(din)
  pre_bram_delay1/10(dout7) -> adc1_7/2(data_in)
  pre_bram_delay1/9(dout6) -> adc1_6/2(data_in)
  accs1/6(dout4) -> pre_bram_delay1/7(din4)
  post_power_delay1/1(sync_out) -> acc_cntrl1/2(sync)
  power1/6(dout4) -> power_convert1/6(din4)
  power_convert1/8(dout6) -> post_power_delay1/8(din6)
  accs1/2(dout0) -> pre_bram_delay1/3(din0)
  adc1_0/1(data_out) -> Terminator9/1
  adc1_1/1(data_out) -> Terminator10/1
  accs1/3(dout1) -> pre_bram_delay1/4(din1)
  accs1/8(dout6) -> pre_bram_delay1/9(din6)
  adc1_7/1(data_out) -> Terminator8/1
  post_power_delay1/8(dout6) -> Delay8/1
  adc1_6/1(data_out) -> Terminator7/1
  power_convert1/7(dout5) -> post_power_delay1/7(din5)
  power1/3(dout1) -> power_convert1/3(din1)
  Delay10/1 -> accs1/4(din2)
  power_convert1/3(dout1) -> post_power_delay1/3(din1)
  Delay7/1 -> accs1/7(din5)
  post_power_delay1/9(dout7) -> Delay9/1
  Delay5/1 -> accs1/3(din1)
  accs1/1(valid) -> counter1/2, pre_bram_delay1/2(valid_in)
  power1/1(sync_out) -> power_convert1/1(sync_in)
  pre_bram_delay1/2(valid_out) -> adc1_7/3(we), adc1_6/3(we), adc1_5/3(we), adc1_4/3(we), adc1_3/3(we), adc1_2/3(we), adc1_1/3(we), adc1_0/3(we)
  power_convert1/1(sync_out) -> post_power_delay1/1(sync_in)
  power_convert1/5(dout3) -> post_power_delay1/5(din3)
  pre_bram_delay1/4(dout1) -> adc1_1/2(data_in)
  adc1_3/1(data_out) -> Terminator12/1
  power_convert1/9(dout7) -> post_power_delay1/9(din7)
  power1/5(dout3) -> power_convert1/5(din3)
  pre_bram_delay1/6(dout3) -> adc1_3/2(data_in)
  [cnt_rst] -> acc_cntrl1/3(rst)
  Delay8/1 -> accs1/8(din6)
  power_convert1/6(dout4) -> post_power_delay1/6(din4)
  adc1_4/1(data_out) -> Terminator1/1
  post_power_delay1/6(dout4) -> Delay6/1
  pre_bram_delay1/3(dout0) -> adc1_0/2(data_in)
  post_power_delay1/7(dout5) -> Delay7/1
  post_power_delay1/4(dout2) -> Delay10/1
  post_power_delay1/3(dout1) -> Delay5/1
  post_power_delay1/5(dout3) -> Delay11/1
  power1/9(dout7) -> power_convert1/9(din7)
  power1/7(dout5) -> power_convert1/7(din5)
  accs1/5(dout3) -> pre_bram_delay1/6(din3)
  pre_bram_delay1/5(dout2) -> adc1_2/2(data_in)
  adc1_2/1(data_out) -> Terminator11/1
  Delay6/1 -> accs1/6(din4)
  pre_bram_delay1/1(addr_out) -> adc1_2/1(addr), adc1_7/1(addr), adc1_6/1(addr), adc1_5/1(addr), adc1_4/1(addr), adc1_3/1(addr), adc1_1/1(addr), adc1_0/1(addr)
  counter1/1 -> pre_bram_delay1/1(addr_in)
  pre_bram_delay1/8(dout5) -> adc1_5/2(data_in)
